simulink model "AcasXuClosedLoop_all_comp"  (Simulink 10.0)
settings: Solver=VariableStepAuto  StartTime=0.0  StopTime=20  RelTol=1e-3
libraries: neural x14040, simulink x2
(part 37 of 534)

== system AcasXuClosedLoop_all_comp/networks/Networks_m_9/AcasXu_2_9/Layer 5 ==
  "a{4} " : Inport  PortDimensions=50
      mask Display: e = 0.06;c1 = [120 230 180]/255;c2 = ([120 230 180]+0)/(256+128);c3 = ([120 230 180]+128)/(256+128);patch([0 1 1 0],[0 0 1 1],c1);patch([0 1 1 1-e 1-e 0+e],[0 0 1 1-e 0+e 0+e],c2);patch([0 0 1 1-e 0+e 0+e],[0 1 1 1-e 1-e 0+e],c3);plot([0 1 1 0 0],[0 0 1 1 0]);
  Delays 1 : Subsystem
      mask Display: e = 0.06;c1 = [120 150 230]/255;c2 = ([120 150 230]+0)/383;c3 = ([120 150 230]+128)/383;patch([0 1 1 0],[0 0 1 1],c1);patch([0 1 1 1-e 1-e 0+e],[0 0 1 1-e 0+e 0+e],c2);patch([0 0 1 1-e 0+e 0+e],[0 1 1 1-e 1-e 0+e],c3);plot([0 1 1 0 0],[0 0 1 1 0]);disp('0');
  "LW{5,4}" : Subsystem
      mask Display: e = 0.06;c1 = [120 150 230]/255;c2 = ([120 150 230]+0)/383;c3 = ([120 150 230]+128)/383;patch([0 1 1 0],[0 0 1 1],c1);patch([0 1 1 1-e 1-e 0+e],[0 0 1 1-e 0+e 0+e],c2);patch([0 0 1 1-e 0+e 0+e],[0 1 1 1-e 1-e 0+e],c3);plot([0 1 1 0 0],[0 0 1 1 0]);disp('W');
  "b{5}" : Constant  Value=[-1.912080000000000001847411112976260483264923095703125;1.2460500000000001019628825815743766725063323974609375;2.856149999999999966604491419275291264057159423828125;-1.15819999999999989626076057902537286281585693359375;-3.26635000000000008668621376273222267627716064453125;-2.868939999999999823643292984343133866786956787109375;-0.322438999999999975631936877107364125549793243408203125;-4.8585200000000003939248927053995430469512939453125;0.00826776000000000073353323415403792751021683216094970703125;1.1786600000000000409983158533577807247638702392578125;1.7016999999999999904076730672386474907398223876953125;0.33088200000000000944311295825173147022724151611328125;3.350420000000000175788272827048785984516143798828125;-3.92804999999999981952214511693455278873443603515625;0.65110299999999998732391759403981268405914306640625;2.038600000000000189714910447946749627590179443359375;0.12242699999999999416200324731107684783637523651123046875;-0.7051979999999999915161197350244037806987762451171875;1.2552600000000000424194013248779810965061187744140625;-0.79386999999999996457944462235900573432445526123046875;-0.045150900000000000755751017322836560197174549102783203125;-0.47827399999999997692867736986954696476459503173828125;-1.86057000000000005712763595511205494403839111328125;2.501650000000000151345602716901339590549468994140625;-2.96544000000000007588596417917869985103607177734375;-0.56893000000000004678923914980259723961353302001953125;-1.4840899999999999092636926434352062642574310302734375;-0.94826799999999999979394260662957094609737396240234375;-0.71766300000000005088196530778077431023120880126953125;0.272494999999999987227994324712199158966541290283203125;0.89623799999999997911714899601065553724765777587890625;3.005290000000000016910917111090384423732757568359375;0.328612999999999988443022402861970476806163787841796875;-0.63532500000000002859934511434403248131275177001953125;-4.05874000000000023646862246096134185791015625;0.52727900000000005320544005371630191802978515625;-1.0708699999999999885602619542623870074748992919921875;0.07364610000000000600284266738526639528572559356689453125;0.260485000000000022080115513745113275945186614990234375;0.558702999999999949665152598754502832889556884765625;0.1783879999999999910187398199923336505889892578125;-2.481310000000000126618715512449853122234344482421875;-3.99228000000000005087485988042317330837249755859375;0.448550999999999977507769699514028616249561309814453125;-1.1849700000000000787991893957951106131076812744140625;3.7723399999999998044586391188204288482666015625;2.011979999999999879634060562239028513431549072265625;1.1504399999999999071320644361549057066440582275390625;-4.28300000000000036237679523765109479427337646484375;-1.9330400000000000915179043659009039402008056640625]
      mask Display: e = 0.06;c1 = [120 150 230]/255;c2 = ([120 150 230]+0)/383;c3 = ([120 150 230]+128)/383;patch([0 1 1 0],[0 0 1 1],c1);patch([0 1 1 1-e 1-e 0+e],[0 0 1 1-e 0+e 0+e],c2);patch([0 0 1 1-e 0+e 0+e],[0 1 1 1-e 1-e 0+e],c3);plot([0 1 1 0 0],[0 0 1 1 0]);disp('b');
  netsum : Sum  Inputs=2  SaturateOnIntegerOverflow=on  InputSameDT=on  OutDataTypeStr=Inherit: Same as first input
      mask Display: e = 0.06;c1 = [120 150 230]/255;c2 = ([120 150 230]+0)/383;c3 = ([120 150 230]+128)/383;patch([0 1 1 0],[0 0 1 1],c1);patch([0 1 1 1-e 1-e 0+e],[0 0 1 1-e 0+e 0+e],c2);patch([0 0 1 1-e 0+e 0+e],[0 1 1 1-e 1-e 0+e],c3);plot([0 1 1 0 0],[0 0 1 1 0]);disp('+');
  poslin : neural/Transfer Functions/poslin
      settings: SourceProductName=Deep Learning Toolbox  SourceProductBaseCode=NN
  "a{5}" : Outport  InitialOutput=[0;0;0;0;0;0;0;0;0;0;0;0;0;0;0;0;0;0;0;0;0;0;0;0;0;0;0;0;0;0;0;0;0;0;0;0;0;0;0;0;0;0;0;0;0;0;0;0;0;0]
      mask Display: e = 0.06;c1 = [120 230 180]/255;c2 = ([120 230 180]+0)/(256+128);c3 = ([120 230 180]+128)/(256+128);patch([0 1 1 0],[0 0 1 1],c1);patch([0 1 1 1-e 1-e 0+e],[0 0 1 1-e 0+e 0+e],c2);patch([0 0 1 1-e 0+e 0+e],[0 1 1 1-e 1-e 0+e],c3);plot([0 1 1 0 0],[0 0 1 1 0]);
  wires:
  poslin/1 -> "a{5}"/1
  netsum/1 -> poslin/1
  "b{5}"/1 -> netsum/2
  "LW{5,4}"/1("lz{5,4}") -> netsum/1
  Delays 1/1("pd{5,4}") -> "LW{5,4}"/1("ad{5,4}")
  "a{4} "/1 -> Delays 1/1("p{5}")

== system AcasXuClosedLoop_all_comp/networks/Networks_m_9/AcasXu_2_9/Layer 6 ==
  "a{5} " : Inport  PortDimensions=50
      mask Display: e = 0.06;c1 = [120 230 180]/255;c2 = ([120 230 180]+0)/(256+128);c3 = ([120 230 180]+128)/(256+128);patch([0 1 1 0],[0 0 1 1],c1);patch([0 1 1 1-e 1-e 0+e],[0 0 1 1-e 0+e 0+e],c2);patch([0 0 1 1-e 0+e 0+e],[0 1 1 1-e 1-e 0+e],c3);plot([0 1 1 0 0],[0 0 1 1 0]);
  Delays 1 : Subsystem
      mask Display: e = 0.06;c1 = [120 150 230]/255;c2 = ([120 150 230]+0)/383;c3 = ([120 150 230]+128)/383;patch([0 1 1 0],[0 0 1 1],c1);patch([0 1 1 1-e 1-e 0+e],[0 0 1 1-e 0+e 0+e],c2);patch([0 0 1 1-e 0+e 0+e],[0 1 1 1-e 1-e 0+e],c3);plot([0 1 1 0 0],[0 0 1 1 0]);disp('0');
  "LW{6,5}" : Subsystem
      mask Display: e = 0.06;c1 = [120 150 230]/255;c2 = ([120 150 230]+0)/383;c3 = ([120 150 230]+128)/383;patch([0 1 1 0],[0 0 1 1],c1);patch([0 1 1 1-e 1-e 0+e],[0 0 1 1-e 0+e 0+e],c2);patch([0 0 1 1-e 0+e 0+e],[0 1 1 1-e 1-e 0+e],c3);plot([0 1 1 0 0],[0 0 1 1 0]);disp('W');
  "b{6}" : Constant  Value=[-0.44546200000000002461320036672987043857574462890625;0.005623999999999999659106020288845684262923896312713623046875;5.890679999999999694182406528852880001068115234375;0.277841000000000004632738637155853211879730224609375;1.4084000000000000962785406954935751855373382568359375;0.0146207999999999997686739305891023832373321056365966796875;3.39046000000000002927436071331612765789031982421875;-5.8207199999999996720134731731377542018890380859375;-7.1486400000000003274180926382541656494140625;4.2595200000000001949729266925714910030364990234375;-5.89017000000000034987124308827333152294158935546875;-6.1283200000000004337152859079651534557342529296875;0.1922750000000000014654943925052066333591938018798828125;0.1213889999999999969038100289253634400665760040283203125;-5.73660000000000014352963262354023754596710205078125;3.083650000000000002131628207280300557613372802734375;0.78354299999999998949107293810811825096607208251953125;-5.58091999999999988091303748660720884799957275390625;-1.2775000000000000799360577730112709105014801025390625;-9.1302000000000003154809746774844825267791748046875;-1.608000000000000095923269327613525092601776123046875;3.3912800000000000721911419532261788845062255859375;-3.998089999999999921698190519236959517002105712890625;1.560869999999999979678477757261134684085845947265625;-10.4304000000000005599076757789589464664459228515625;-8.982169999999999987494447850622236728668212890625;-9.91011999999999915189619059674441814422607421875;-4.880630000000000023874235921539366245269775390625;-1.470450000000000034816594052244909107685089111328125;-3.563989999999999991331378623726777732372283935546875;-4.8996399999999997731947587453760206699371337890625;-6.07057999999999964302332955412566661834716796875;1.1889600000000000168398628375143744051456451416015625;0.056920900000000003438049844817214761860668659210205078125;-3.316940000000000221547225009999237954616546630859375;-5.3012899999999998357225194922648370265960693359375;-0.0309107999999999986939780427519508521072566509246826171875;-7.2179000000000002046363078989088535308837890625;3.530860000000000109565689854207448661327362060546875;-12.779199999999999448618837050162255764007568359375;-3.3554300000000001347189027001149952411651611328125;0.11294099999999999972555286831266130320727825164794921875;3.32683000000000017593038137420080602169036865234375;-6.00711999999999957111640469520352780818939208984375;1.030790000000000095070618044701404869556427001953125;4.65157000000000042660985855036415159702301025390625;-7.62272999999999978371079123462550342082977294921875;1.97126000000000001222133505507372319698333740234375;-0.984769000000000005456968210637569427490234375;-9.0827399999999993696064848336391150951385498046875]
      mask Display: e = 0.06;c1 = [120 150 230]/255;c2 = ([120 150 230]+0)/383;c3 = ([120 150 230]+128)/383;patch([0 1 1 0],[0 0 1 1],c1);patch([0 1 1 1-e 1-e 0+e],[0 0 1 1-e 0+e 0+e],c2);patch([0 0 1 1-e 0+e 0+e],[0 1 1 1-e 1-e 0+e],c3);plot([0 1 1 0 0],[0 0 1 1 0]);disp('b');
  netsum : Sum  Inputs=2  SaturateOnIntegerOverflow=on  InputSameDT=on  OutDataTypeStr=Inherit: Same as first input
      mask Display: e = 0.06;c1 = [120 150 230]/255;c2 = ([120 150 230]+0)/383;c3 = ([120 150 230]+128)/383;patch([0 1 1 0],[0 0 1 1],c1);patch([0 1 1 1-e 1-e 0+e],[0 0 1 1-e 0+e 0+e],c2);patch([0 0 1 1-e 0+e 0+e],[0 1 1 1-e 1-e 0+e],c3);plot([0 1 1 0 0],[0 0 1 1 0]);disp('+');
  poslin : neural/Transfer Functions/poslin
      settings: SourceProductName=Deep Learning Toolbox  SourceProductBaseCode=NN
  "a{6}" : Outport  InitialOutput=[0;0;0;0;0;0;0;0;0;0;0;0;0;0;0;0;0;0;0;0;0;0;0;0;0;0;0;0;0;0;0;0;0;0;0;0;0;0;0;0;0;0;0;0;0;0;0;0;0;0]
      mask Display: e = 0.06;c1 = [120 230 180]/255;c2 = ([120 230 180]+0)/(256+128);c3 = ([120 230 180]+128)/(256+128);patch([0 1 1 0],[0 0 1 1],c1);patch([0 1 1 1-e 1-e 0+e],[0 0 1 1-e 0+e 0+e],c2);patch([0 0 1 1-e 0+e 0+e],[0 1 1 1-e 1-e 0+e],c3);plot([0 1 1 0 0],[0 0 1 1 0]);
  wires:
  poslin/1 -> "a{6}"/1
  netsum/1 -> poslin/1
  "b{6}"/1 -> netsum/2
  "LW{6,5}"/1("lz{6,5}") -> netsum/1
  Delays 1/1("pd{6,5}") -> "LW{6,5}"/1("ad{6,5}")
  "a{5} "/1 -> Delays 1/1("p{6}")

== system AcasXuClosedLoop_all_comp/networks/Networks_m_9/AcasXu_2_9/Layer 7 ==
  "a{6} " : Inport  PortDimensions=50
      mask Display: e = 0.06;c1 = [120 230 180]/255;c2 = ([120 230 180]+0)/(256+128);c3 = ([120 230 180]+128)/(256+128);patch([0 1 1 0],[0 0 1 1],c1);patch([0 1 1 1-e 1-e 0+e],[0 0 1 1-e 0+e 0+e],c2);patch([0 0 1 1-e 0+e 0+e],[0 1 1 1-e 1-e 0+e],c3);plot([0 1 1 0 0],[0 0 1 1 0]);
  Delays 1 : Subsystem
      mask Display: e = 0.06;c1 = [120 150 230]/255;c2 = ([120 150 230]+0)/383;c3 = ([120 150 230]+128)/383;patch([0 1 1 0],[0 0 1 1],c1);patch([0 1 1 1-e 1-e 0+e],[0 0 1 1-e 0+e 0+e],c2);patch([0 0 1 1-e 0+e 0+e],[0 1 1 1-e 1-e 0+e],c3);plot([0 1 1 0 0],[0 0 1 1 0]);disp('0');
  "LW{7,6}" : Subsystem
      mask Display: e = 0.06;c1 = [120 150 230]/255;c2 = ([120 150 230]+0)/383;c3 = ([120 150 230]+128)/383;patch([0 1 1 0],[0 0 1 1],c1);patch([0 1 1 1-e 1-e 0+e],[0 0 1 1-e 0+e 0+e],c2);patch([0 0 1 1-e 0+e 0+e],[0 1 1 1-e 1-e 0+e],c3);plot([0 1 1 0 0],[0 0 1 1 0]);disp('W');
  "b{7}" : Constant  Value=[-0.021078199999999998326405403759054024703800678253173828125;-0.0197138000000000000733191285462453379295766353607177734375;0.0182673999999999996102229005146000417880713939666748046875;-0.0185578999999999987025045555810720543377101421356201171875;0.0182602000000000007362555010104188113473355770111083984375]
      mask Display: e = 0.06;c1 = [120 150 230]/255;c2 = ([120 150 230]+0)/383;c3 = ([120 150 230]+128)/383;patch([0 1 1 0],[0 0 1 1],c1);patch([0 1 1 1-e 1-e 0+e],[0 0 1 1-e 0+e 0+e],c2);patch([0 0 1 1-e 0+e 0+e],[0 1 1 1-e 1-e 0+e],c3);plot([0 1 1 0 0],[0 0 1 1 0]);disp('b');
  netsum : Sum  Inputs=2  SaturateOnIntegerOverflow=on  InputSameDT=on  OutDataTypeStr=Inherit: Same as first input
      mask Display: e = 0.06;c1 = [120 150 230]/255;c2 = ([120 150 230]+0)/383;c3 = ([120 150 230]+128)/383;patch([0 1 1 0],[0 0 1 1],c1);patch([0 1 1 1-e 1-e 0+e],[0 0 1 1-e 0+e 0+e],c2);patch([0 0 1 1-e 0+e 0+e],[0 1 1 1-e 1-e 0+e],c3);plot([0 1 1 0 0],[0 0 1 1 0]);disp('+');
  purelin : neural/Transfer Functions/purelin
      settings: SourceProductName=Deep Learning Toolbox
  "a{7}" : Outport  InitialOutput=[0;0;0;0;0]
      mask Display: e = 0.06;c1 = [120 230 180]/255;c2 = ([120 230 180]+0)/(256+128);c3 = ([120 230 180]+128)/(256+128);patch([0 1 1 0],[0 0 1 1],c1);patch([0 1 1 1-e 1-e 0+e],[0 0 1 1-e 0+e 0+e],c2);patch([0 0 1 1-e 0+e 0+e],[0 1 1 1-e 1-e 0+e],c3);plot([0 1 1 0 0],[0 0 1 1 0]);
  wires:
  purelin/1 -> "a{7}"/1
  netsum/1 -> purelin/1
  "b{7}"/1 -> netsum/2
  "LW{7,6}"/1("lz{7,6}") -> netsum/1
  Delays 1/1("pd{7,6}") -> "LW{7,6}"/1("ad{7,6}")
  "a{6} "/1 -> Delays 1/1("p{7}")

== system AcasXuClosedLoop_all_comp/networks/Networks_m_9/AcasXu_2_9/Process Input 1 ==
  x : Inport  PortDimensions=5
      mask Display: e = 0.06;c1 = [120 230 180]/255;c2 = ([120 230 180]+0)/(256+128);c3 = ([120 230 180]+128)/(256+128);patch([0 1 1 0],[0 0 1 1],c1);patch([0 1 1 1-e 1-e 0+e],[0 0 1 1-e 0+e 0+e],c2);patch([0 0 1 1-e 0+e 0+e],[0 1 1 1-e 1-e 0+e],c3);plot([0 1 1 0 0],[0 0 1 1 0]);
  p : Outport  PortDimensions=5
      mask Display: e = 0.06;c1 = [120 230 180]/255;c2 = ([120 230 180]+0)/(256+128);c3 = ([120 230 180]+128)/(256+128);patch([0 1 1 0],[0 0 1 1],c1);patch([0 1 1 1-e 1-e 0+e],[0 0 1 1-e 0+e 0+e],c2);patch([0 0 1 1-e 0+e 0+e],[0 1 1 1-e 1-e 0+e],c3);plot([0 1 1 0 0],[0 0 1 1 0]);
  wires:
  x/1 -> p/1

== system AcasXuClosedLoop_all_comp/networks/Networks_m_9/AcasXu_2_9/Process Output 1 ==
  a : Inport  PortDimensions=5
      mask Display: e = 0.06;c1 = [120 230 180]/255;c2 = ([120 230 180]+0)/(256+128);c3 = ([120 230 180]+128)/(256+128);patch([0 1 1 0],[0 0 1 1],c1);patch([0 1 1 1-e 1-e 0+e],[0 0 1 1-e 0+e 0+e],c2);patch([0 0 1 1-e 0+e 0+e],[0 1 1 1-e 1-e 0+e],c3);plot([0 1 1 0 0],[0 0 1 1 0]);
  y : Outport  PortDimensions=5
      mask Display: e = 0.06;c1 = [120 230 180]/255;c2 = ([120 230 180]+0)/(256+128);c3 = ([120 230 180]+128)/(256+128);patch([0 1 1 0],[0 0 1 1],c1);patch([0 1 1 1-e 1-e 0+e],[0 0 1 1-e 0+e 0+e],c2);patch([0 0 1 1-e 0+e 0+e],[0 1 1 1-e 1-e 0+e],c3);plot([0 1 1 0 0],[0 0 1 1 0]);
  wires:
  a/1 -> y/1

== system AcasXuClosedLoop_all_comp/networks/Networks_m_9/AcasXu_3_9/Layer 1 ==
  "p{1}" : Inport  PortDimensions=5
      mask Display: e = 0.06;c1 = [120 230 180]/255;c2 = ([120 230 180]+0)/(256+128);c3 = ([120 230 180]+128)/(256+128);patch([0 1 1 0],[0 0 1 1],c1);patch([0 1 1 1-e 1-e 0+e],[0 0 1 1-e 0+e 0+e],c2);patch([0 0 1 1-e 0+e 0+e],[0 1 1 1-e 1-e 0+e],c3);plot([0 1 1 0 0],[0 0 1 1 0]);
  Delays 1 : Subsystem
      mask Display: e = 0.06;c1 = [120 150 230]/255;c2 = ([120 150 230]+0)/383;c3 = ([120 150 230]+128)/383;patch([0 1 1 0],[0 0 1 1],c1);patch([0 1 1 1-e 1-e 0+e],[0 0 1 1-e 0+e 0+e],c2);patch([0 0 1 1-e 0+e 0+e],[0 1 1 1-e 1-e 0+e],c3);plot([0 1 1 0 0],[0 0 1 1 0]);disp('0');
  "IW{1,1}" : Subsystem
      mask Display: e = 0.06;c1 = [120 150 230]/255;c2 = ([120 150 230]+0)/383;c3 = ([120 150 230]+128)/383;patch([0 1 1 0],[0 0 1 1],c1);patch([0 1 1 1-e 1-e 0+e],[0 0 1 1-e 0+e 0+e],c2);patch([0 0 1 1-e 0+e 0+e],[0 1 1 1-e 1-e 0+e],c3);plot([0 1 1 0 0],[0 0 1 1 0]);disp('W');
  "b{1}" : Constant  Value=[0.030622900000000001508038138808842631988227367401123046875;0.2441520000000000079065642921705148182809352874755859375;0.0133717999999999996585398065462868544273078441619873046875;0.028875299999999999578381704168350552208721637725830078125;-0.53588299999999999823074858795735053718090057373046875;-0.1026019999999999987583265692592249251902103424072265625;-0.6446739999999999692903429604484699666500091552734375;-0.44969999999999998863131622783839702606201171875;-0.0275753999999999999115374293978675268590450286865234375;-0.021906899999999999983923970603427733294665813446044921875;-0.01108540000000000046165293809963259263895452022552490234375;-0.7542370000000000462847538074129261076450347900390625;0.2315709999999999990638599456360680051147937774658203125;0.6522229999999999972004616211052052676677703857421875;-0.00140032999999999997753352687368533224798738956451416015625;-0.54398000000000001907807245515868999063968658447265625;-0.041275499999999999689581642314806231297552585601806640625;-0.49519000000000001904254531837068498134613037109375;-0.2935820000000000096207486421917565166950225830078125;-0.52670300000000003226574563086614944040775299072265625;-0.022206599999999999950350826338762999512255191802978515625;-0.377709999999999990194510246510617434978485107421875;0.06179679999999999895354818590931245125830173492431640625;0.2306709999999999871622691216543898917734622955322265625;-0.395853999999999983661069791196496225893497467041015625;0.0292625999999999997225330616856808774173259735107421875;0.1778720000000000023288038164537283591926097869873046875;-0.09989320000000000143725031875874265097081661224365234375;-0.5966599999999999681676854379475116729736328125;0.2298300000000000065103478164019179530441761016845703125;-0.2032980000000000064819261069715139456093311309814453125;0.110547000000000006370015626089298166334629058837890625;0.10639600000000000445954384531432879157364368438720703125;-0.1522880000000000066950889276995440013706684112548828125;-0.027030800000000000660183019363103085197508335113525390625;0.120275999999999994027888305936357937753200531005859375;0.039729300000000002113242913992507965303957462310791015625;0.10090399999999999369748593380791135132312774658203125;0.0299061000000000014320544750034969183616340160369873046875;0.1286940000000000028368418725221999920904636383056640625;-0.09250629999999999963922192591780913062393665313720703125;0.00146498000000000005819955628538764358381740748882293701171875;-0.565617000000000036408209780347533524036407470703125;-0.02543890000000000040092373865263652987778186798095703125;-0.019948899999999998577937532218129490502178668975830078125;-0.10592500000000000526245713672324200160801410675048828125;-0.390361000000000013532286402551108039915561676025390625;-0.2574980000000000046611603465862572193145751953125;-0.1269470000000000042827963397940038703382015228271484375;-0.23213200000000000500222085975110530853271484375]
      mask Display: e = 0.06;c1 = [120 150 230]/255;c2 = ([120 150 230]+0)/383;c3 = ([120 150 230]+128)/383;patch([0 1 1 0],[0 0 1 1],c1);patch([0 1 1 1-e 1-e 0+e],[0 0 1 1-e 0+e 0+e],c2);patch([0 0 1 1-e 0+e 0+e],[0 1 1 1-e 1-e 0+e],c3);plot([0 1 1 0 0],[0 0 1 1 0]);disp('b');
  netsum : Sum  Inputs=2  SaturateOnIntegerOverflow=on  InputSameDT=on  OutDataTypeStr=Inherit: Same as first input
      mask Display: e = 0.06;c1 = [120 150 230]/255;c2 = ([120 150 230]+0)/383;c3 = ([120 150 230]+128)/383;patch([0 1 1 0],[0 0 1 1],c1);patch([0 1 1 1-e 1-e 0+e],[0 0 1 1-e 0+e 0+e],c2);patch([0 0 1 1-e 0+e 0+e],[0 1 1 1-e 1-e 0+e],c3);plot([0 1 1 0 0],[0 0 1 1 0]);disp('+');
  poslin : neural/Transfer Functions/poslin
      settings: SourceProductName=Deep Learning Toolbox  SourceProductBaseCode=NN
  "a{1}" : Outport  InitialOutput=[0;0;0;0;0;0;0;0;0;0;0;0;0;0;0;0;0;0;0;0;0;0;0;0;0;0;0;0;0;0;0;0;0;0;0;0;0;0;0;0;0;0;0;0;0;0;0;0;0;0]
      mask Display: e = 0.06;c1 = [120 230 180]/255;c2 = ([120 230 180]+0)/(256+128);c3 = ([120 230 180]+128)/(256+128);patch([0 1 1 0],[0 0 1 1],c1);patch([0 1 1 1-e 1-e 0+e],[0 0 1 1-e 0+e 0+e],c2);patch([0 0 1 1-e 0+e 0+e],[0 1 1 1-e 1-e 0+e],c3);plot([0 1 1 0 0],[0 0 1 1 0]);
  wires:
  poslin/1 -> "a{1}"/1
  netsum/1 -> poslin/1
  "b{1}"/1 -> netsum/2
  "IW{1,1}"/1("iz{1,1}") -> netsum/1
  Delays 1/1("pd{1,1}") -> "IW{1,1}"/1("pd{1,1}")
  "p{1}"/1 -> Delays 1/1("p{1}")

== system AcasXuClosedLoop_all_comp/networks/Networks_m_9/AcasXu_3_9/Layer 2 ==
  "a{1} " : Inport  PortDimensions=50
      mask Display: e = 0.06;c1 = [120 230 180]/255;c2 = ([120 230 180]+0)/(256+128);c3 = ([120 230 180]+128)/(256+128);patch([0 1 1 0],[0 0 1 1],c1);patch([0 1 1 1-e 1-e 0+e],[0 0 1 1-e 0+e 0+e],c2);patch([0 0 1 1-e 0+e 0+e],[0 1 1 1-e 1-e 0+e],c3);plot([0 1 1 0 0],[0 0 1 1 0]);
  Delays 1 : Subsystem
      mask Display: e = 0.06;c1 = [120 150 230]/255;c2 = ([120 150 230]+0)/383;c3 = ([120 150 230]+128)/383;patch([0 1 1 0],[0 0 1 1],c1);patch([0 1 1 1-e 1-e 0+e],[0 0 1 1-e 0+e 0+e],c2);patch([0 0 1 1-e 0+e 0+e],[0 1 1 1-e 1-e 0+e],c3);plot([0 1 1 0 0],[0 0 1 1 0]);disp('0');
  "LW{2,1}" : Subsystem
      mask Display: e = 0.06;c1 = [120 150 230]/255;c2 = ([120 150 230]+0)/383;c3 = ([120 150 230]+128)/383;patch([0 1 1 0],[0 0 1 1],c1);patch([0 1 1 1-e 1-e 0+e],[0 0 1 1-e 0+e 0+e],c2);patch([0 0 1 1-e 0+e 0+e],[0 1 1 1-e 1-e 0+e],c3);plot([0 1 1 0 0],[0 0 1 1 0]);disp('W');
  "b{2}" : Constant  Value=[1.1304700000000000859046167533961124718189239501953125;-0.020277599999999999791366889212440582923591136932373046875;1.5884499999999999175770426518283784389495849609375;-1.934600000000000097344354799133725464344024658203125;-0.0215263000000000016831425142527223215438425540924072265625;-1.02658000000000004803268893738277256488800048828125;0.37367099999999997539390506062773056328296661376953125;-0.0209797000000000004316103030532758566550910472869873046875;0.13788400000000000655830945106572471559047698974609375;0.9806209999999999649133997081662528216838836669921875;1.7327600000000000779465381128829903900623321533203125;-0.046625199999999998590194394409991218708455562591552734375;0.290381999999999973471886960396659560501575469970703125;-0.403724000000000027288393766866647638380527496337890625;-0.0184929999999999990223376045150871505029499530792236328125;-0.0272172999999999998266275724745355546474456787109375;-0.036036800000000000776534392343819490633904933929443359375;-0.021002500000000000335287353436797275207936763763427734375;-0.032667099999999997528288275816521490924060344696044921875;-0.0185414999999999989543919554080275702290236949920654296875;-1.3001899999999999568700559393619187176227569580078125;1.0247599999999998932054268152569420635700225830078125;-0.0304184000000000016872281349833428976126015186309814453125;-0.52067600000000002768985041257110424339771270751953125;0.306744999999999989892529583812574855983257293701171875;0.2081530000000000046878056991772609762847423553466796875;-0.01226089999999999992363886036628173314966261386871337890625;-0.013461199999999999554756158204327221028506755828857421875;-0.0311606000000000001037836483419596333988010883331298828125;-0.020854799999999999726707500258271466009318828582763671875;-0.0218689000000000001444622199642253690399229526519775390625;0.89031099999999996352784137343405745923519134521484375;-0.0130860999999999998155697511492689955048263072967529296875;-0.0115549999999999992994492714615262229926884174346923828125;1.01635000000000008668621376273222267627716064453125;-0.29596699999999998009769797135959379374980926513671875;1.4970399999999999263167183016776107251644134521484375;-0.4670900000000000051869619710487313568592071533203125;-0.0189642999999999999072297640623219194822013378143310546875;-0.0109682000000000008654854610767870326526463031768798828125;-0.21932099999999998818367430430953390896320343017578125;-1.5291900000000000492406115881749428808689117431640625;-0.01226900000000000039157566078529271180741488933563232421875;-0.20906700000000000283506551568279974162578582763671875;0.74215299999999995161914512209477834403514862060546875;-0.01379310000000000084596774030387678067199885845184326171875;-0.01690679999999999960635932438890449702739715576171875;-0.37743300000000001848121655712020583450794219970703125;-0.0114754999999999995896615700985421426594257354736328125;0.738626000000000004774847184307873249053955078125]
      mask Display: e = 0.06;c1 = [120 150 230]/255;c2 = ([120 150 230]+0)/383;c3 = ([120 150 230]+128)/383;patch([0 1 1 0],[0 0 1 1],c1);patch([0 1 1 1-e 1-e 0+e],[0 0 1 1-e 0+e 0+e],c2);patch([0 0 1 1-e 0+e 0+e],[0 1 1 1-e 1-e 0+e],c3);plot([0 1 1 0 0],[0 0 1 1 0]);disp('b');
  netsum : Sum  Inputs=2  SaturateOnIntegerOverflow=on  InputSameDT=on  OutDataTypeStr=Inherit: Same as first input
      mask Display: e = 0.06;c1 = [120 150 230]/255;c2 = ([120 150 230]+0)/383;c3 = ([120 150 230]+128)/383;patch([0 1 1 0],[0 0 1 1],c1);patch([0 1 1 1-e 1-e 0+e],[0 0 1 1-e 0+e 0+e],c2);patch([0 0 1 1-e 0+e 0+e],[0 1 1 1-e 1-e 0+e],c3);plot([0 1 1 0 0],[0 0 1 1 0]);disp('+');
  poslin : neural/Transfer Functions/poslin
      settings: SourceProductName=Deep Learning Toolbox  SourceProductBaseCode=NN
  "a{2}" : Outport  InitialOutput=[0;0;0;0;0;0;0;0;0;0;0;0;0;0;0;0;0;0;0;0;0;0;0;0;0;0;0;0;0;0;0;0;0;0;0;0;0;0;0;0;0;0;0;0;0;0;0;0;0;0]
      mask Display: e = 0.06;c1 = [120 230 180]/255;c2 = ([120 230 180]+0)/(256+128);c3 = ([120 230 180]+128)/(256+128);patch([0 1 1 0],[0 0 1 1],c1);patch([0 1 1 1-e 1-e 0+e],[0 0 1 1-e 0+e 0+e],c2);patch([0 0 1 1-e 0+e 0+e],[0 1 1 1-e 1-e 0+e],c3);plot([0 1 1 0 0],[0 0 1 1 0]);
  wires:
  poslin/1 -> "a{2}"/1
  netsum/1 -> poslin/1
  "b{2}"/1 -> netsum/2
  "LW{2,1}"/1("lz{2,1}") -> netsum/1
  Delays 1/1("pd{2,1}") -> "LW{2,1}"/1("ad{2,1}")
  "a{1} "/1 -> Delays 1/1("p{2}")

== system AcasXuClosedLoop_all_comp/networks/Networks_m_9/AcasXu_3_9/Layer 3 ==
  "a{2} " : Inport  PortDimensions=50
      mask Display: e = 0.06;c1 = [120 230 180]/255;c2 = ([120 230 180]+0)/(256+128);c3 = ([120 230 180]+128)/(256+128);patch([0 1 1 0],[0 0 1 1],c1);patch([0 1 1 1-e 1-e 0+e],[0 0 1 1-e 0+e 0+e],c2);patch([0 0 1 1-e 0+e 0+e],[0 1 1 1-e 1-e 0+e],c3);plot([0 1 1 0 0],[0 0 1 1 0]);
  Delays 1 : Subsystem
      mask Display: e = 0.06;c1 = [120 150 230]/255;c2 = ([120 150 230]+0)/383;c3 = ([120 150 230]+128)/383;patch([0 1 1 0],[0 0 1 1],c1);patch([0 1 1 1-e 1-e 0+e],[0 0 1 1-e 0+e 0+e],c2);patch([0 0 1 1-e 0+e 0+e],[0 1 1 1-e 1-e 0+e],c3);plot([0 1 1 0 0],[0 0 1 1 0]);disp('0');
  "LW{3,2}" : Subsystem
      mask Display: e = 0.06;c1 = [120 150 230]/255;c2 = ([120 150 230]+0)/383;c3 = ([120 150 230]+128)/383;patch([0 1 1 0],[0 0 1 1],c1);patch([0 1 1 1-e 1-e 0+e],[0 0 1 1-e 0+e 0+e],c2);patch([0 0 1 1-e 0+e 0+e],[0 1 1 1-e 1-e 0+e],c3);plot([0 1 1 0 0],[0 0 1 1 0]);disp('W');
  "b{3}" : Constant  Value=[-0.94015199999999998770050524399266578257083892822265625;-0.57625300000000001521271997262374497950077056884765625;0.13505800000000001137578919951920397579669952392578125;-0.95997399999999999398170302811195142567157745361328125;-0.405694999999999972306596873750095255672931671142578125;0.28235799999999999787547722007730044424533843994140625;0.55059599999999997432809095698758028447628021240234375;-0.0190257999999999989626520147112387348897755146026611328125;-0.0220898999999999991195043236302808509208261966705322265625;-0.94089199999999995061017443731543608009815216064453125;0.035043100000000000637800923186659929342567920684814453125;-0.186477000000000003865352482534945011138916015625;-0.360105999999999981664444703710614703595638275146484375;-0.56810700000000002862776682377443648874759674072265625;1.3017700000000000937205868467572145164012908935546875;-0.70688099999999998157562686174060218036174774169921875;0.325824999999999975752729142186581157147884368896484375;-0.8053090000000000525659515915322117507457733154296875;-0.417132000000000002781774810500792227685451507568359375;-0.327361999999999986332710477654472924768924713134765625;-1.4274299999999999766231439934927038848400115966796875;0.052068200000000001981259600825069355778396129608154296875;-1.2055499999999998994582028899458236992359161376953125;1.8280600000000000182609483090345747768878936767578125;-0.046557700000000000473132644174256711266934871673583984375;-0.039566600000000000381117359893323737196624279022216796875;-0.7274030000000000217852402784046716988086700439453125;-1.2236100000000000864730509420041926205158233642578125;-1.490320000000000089102059064316563308238983154296875;0.09272080000000000599147398361310479231178760528564453125;0.52890000000000003677058657558518461883068084716796875;-0.9478429999999999910897940935683436691761016845703125;-0.62088799999999999545963191849295981228351593017578125;0.672177000000000024471091819577850401401519775390625;-0.6047759999999999802611228005844168365001678466796875;-1.104600000000000026290081223123706877231597900390625;-0.368530999999999997474020574372843839228153228759765625;-0.86718499999999998362198994072969071567058563232421875;-0.2483660000000000034336977705606841482222080230712890625;-0.0255894000000000017280843422895486583001911640167236328125;1.93771000000000004348521542851813137531280517578125;0.99385500000000004394706820676219649612903594970703125;-0.34159600000000001074340616469271481037139892578125;-0.006038739999999999906454828391133560216985642910003662109375;-0.2003359999999999863096178387422696687281131744384765625;0.1015790000000000026236790517941699363291263580322265625;-0.0100161000000000000031974423109204508364200592041015625;-0.5780790000000000095070618044701404869556427001953125;-0.91156800000000004491340632739593274891376495361328125;-1.043930000000000024584778657299466431140899658203125]
      mask Display: e = 0.06;c1 = [120 150 230]/255;c2 = ([120 150 230]+0)/383;c3 = ([120 150 230]+128)/383;patch([0 1 1 0],[0 0 1 1],c1);patch([0 1 1 1-e 1-e 0+e],[0 0 1 1-e 0+e 0+e],c2);patch([0 0 1 1-e 0+e 0+e],[0 1 1 1-e 1-e 0+e],c3);plot([0 1 1 0 0],[0 0 1 1 0]);disp('b');
  netsum : Sum  Inputs=2  SaturateOnIntegerOverflow=on  InputSameDT=on  OutDataTypeStr=Inherit: Same as first input
      mask Display: e = 0.06;c1 = [120 150 230]/255;c2 = ([120 150 230]+0)/383;c3 = ([120 150 230]+128)/383;patch([0 1 1 0],[0 0 1 1],c1);patch([0 1 1 1-e 1-e 0+e],[0 0 1 1-e 0+e 0+e],c2);patch([0 0 1 1-e 0+e 0+e],[0 1 1 1-e 1-e 0+e],c3);plot([0 1 1 0 0],[0 0 1 1 0]);disp('+');
  poslin : neural/Transfer Functions/poslin
      settings: SourceProductName=Deep Learning Toolbox  SourceProductBaseCode=NN
  "a{3}" : Outport  InitialOutput=[0;0;0;0;0;0;0;0;0;0;0;0;0;0;0;0;0;0;0;0;0;0;0;0;0;0;0;0;0;0;0;0;0;0;0;0;0;0;0;0;0;0;0;0;0;0;0;0;0;0]
      mask Display: e = 0.06;c1 = [120 230 180]/255;c2 = ([120 230 180]+0)/(256+128);c3 = ([120 230 180]+128)/(256+128);patch([0 1 1 0],[0 0 1 1],c1);patch([0 1 1 1-e 1-e 0+e],[0 0 1 1-e 0+e 0+e],c2);patch([0 0 1 1-e 0+e 0+e],[0 1 1 1-e 1-e 0+e],c3);plot([0 1 1 0 0],[0 0 1 1 0]);
  wires:
  poslin/1 -> "a{3}"/1
  netsum/1 -> poslin/1
  "b{3}"/1 -> netsum/2
  "LW{3,2}"/1("lz{3,2}") -> netsum/1
  Delays 1/1("pd{3,2}") -> "LW{3,2}"/1("ad{3,2}")
  "a{2} "/1 -> Delays 1/1("p{3}")

== system AcasXuClosedLoop_all_comp/networks/Networks_m_9/AcasXu_3_9/Layer 4 ==
  "a{3} " : Inport  PortDimensions=50
      mask Display: e = 0.06;c1 = [120 230 180]/255;c2 = ([120 230 180]+0)/(256+128);c3 = ([120 230 180]+128)/(256+128);patch([0 1 1 0],[0 0 1 1],c1);patch([0 1 1 1-e 1-e 0+e],[0 0 1 1-e 0+e 0+e],c2);patch([0 0 1 1-e 0+e 0+e],[0 1 1 1-e 1-e 0+e],c3);plot([0 1 1 0 0],[0 0 1 1 0]);
  Delays 1 : Subsystem
      mask Display: e = 0.06;c1 = [120 150 230]/255;c2 = ([120 150 230]+0)/383;c3 = ([120 150 230]+128)/383;patch([0 1 1 0],[0 0 1 1],c1);patch([0 1 1 1-e 1-e 0+e],[0 0 1 1-e 0+e 0+e],c2);patch([0 0 1 1-e 0+e 0+e],[0 1 1 1-e 1-e 0+e],c3);plot([0 1 1 0 0],[0 0 1 1 0]);disp('0');
  "LW{4,3}" : Subsystem
      mask Display: e = 0.06;c1 = [120 150 230]/255;c2 = ([120 150 230]+0)/383;c3 = ([120 150 230]+128)/383;patch([0 1 1 0],[0 0 1 1],c1);patch([0 1 1 1-e 1-e 0+e],[0 0 1 1-e 0+e 0+e],c2);patch([0 0 1 1-e 0+e 0+e],[0 1 1 1-e 1-e 0+e],c3);plot([0 1 1 0 0],[0 0 1 1 0]);disp('W');
  "b{4}" : Constant  Value=[-1.7806699999999999750599499748204834759235382080078125;-1.37105999999999994543031789362430572509765625;-2.34382000000000001449507180950604379177093505859375;0.50524299999999999766231439934927038848400115966796875;-3.99293999999999993377741702715866267681121826171875;3.939529999999999976267872625612653791904449462890625;-1.6768400000000001082156586562632583081722259521484375;-0.021833499999999998741895268494772608391940593719482421875;1.2009600000000000274980038739158771932125091552734375;0.41558499999999998220090446920949034392833709716796875;3.70685999999999982179588187136687338352203369140625;-1.44794000000000000483169060316868126392364501953125;1.147559999999999913455894784419797360897064208984375;-1.581539999999999945856643535080365836620330810546875;1.3639900000000000357402996087330393493175506591796875;0.786737000000000019639401216409169137477874755859375;-3.29525999999999985590193318785168230533599853515625;-0.0174671999999999986996623846380316535942256450653076171875;0.2804729999999999723314658695016987621784210205078125;-2.61798000000000019582557797548361122608184814453125;0.435118999999999978012255041903699748218059539794921875;0.922158000000000033224978324142284691333770751953125;-1.2847999999999999420907670355518348515033721923828125;1.555839999999999889723767410032451152801513671875;3.292829999999999923687710179365240037441253662109375;-0.615458999999999978314235704601742327213287353515625;1.085129999999999927950966593925841152667999267578125;-0.2290149999999999963495866950324852950870990753173828125;-1.8910899999999999376854020738392136991024017333984375;-0.7731500000000000039079850466805510222911834716796875;-0.97648900000000005139355607752804644405841827392578125;0.426566999999999973969266875428729690611362457275390625;-2.37408999999999981156406647642143070697784423828125;-1.9548200000000000020605739337042905390262603759765625;1.6051800000000000512301312483032234013080596923828125;4.26851999999999964785502015729434788227081298828125;0.470463000000000020062174144186428748071193695068359375;-0.07553169999999999328910149642979376949369907379150390625;0.5801410000000000177777792487177066504955291748046875;1.7715899999999999980815346134477294981479644775390625;-2.001310000000000144382283906452357769012451171875;-0.006227690000000000135338407147855832590721547603607177734375;-1.2850200000000000510880227011512033641338348388671875;1.6148800000000000931521526581491343677043914794921875;-1.2901499999999999079847157190670259296894073486328125;0.1793760000000000076614270483332802541553974151611328125;-0.43104500000000001147526518252561800181865692138671875;0.0135645999999999995744293102006849949248135089874267578125;-0.62482300000000001727329390632803551852703094482421875;-0.006054269999999999617157353526408769539557397365570068359375]
      mask Display: e = 0.06;c1 = [120 150 230]/255;c2 = ([120 150 230]+0)/383;c3 = ([120 150 230]+128)/383;patch([0 1 1 0],[0 0 1 1],c1);patch([0 1 1 1-e 1-e 0+e],[0 0 1 1-e 0+e 0+e],c2);patch([0 0 1 1-e 0+e 0+e],[0 1 1 1-e 1-e 0+e],c3);plot([0 1 1 0 0],[0 0 1 1 0]);disp('b');
  netsum : Sum  Inputs=2  SaturateOnIntegerOverflow=on  InputSameDT=on  OutDataTypeStr=Inherit: Same as first input
      mask Display: e = 0.06;c1 = [120 150 230]/255;c2 = ([120 150 230]+0)/383;c3 = ([120 150 230]+128)/383;patch([0 1 1 0],[0 0 1 1],c1);patch([0 1 1 1-e 1-e 0+e],[0 0 1 1-e 0+e 0+e],c2);patch([0 0 1 1-e 0+e 0+e],[0 1 1 1-e 1-e 0+e],c3);plot([0 1 1 0 0],[0 0 1 1 0]);disp('+');
  poslin : neural/Transfer Functions/poslin
      settings: SourceProductName=Deep Learning Toolbox  SourceProductBaseCode=NN
  "a{4}" : Outport  InitialOutput=[0;0;0;0;0;0;0;0;0;0;0;0;0;0;0;0;0;0;0;0;0;0;0;0;0;0;0;0;0;0;0;0;0;0;0;0;0;0;0;0;0;0;0;0;0;0;0;0;0;0]
      mask Display: e = 0.06;c1 = [120 230 180]/255;c2 = ([120 230 180]+0)/(256+128);c3 = ([120 230 180]+128)/(256+128);patch([0 1 1 0],[0 0 1 1],c1);patch([0 1 1 1-e 1-e 0+e],[0 0 1 1-e 0+e 0+e],c2);patch([0 0 1 1-e 0+e 0+e],[0 1 1 1-e 1-e 0+e],c3);plot([0 1 1 0 0],[0 0 1 1 0]);
  wires:
  poslin/1 -> "a{4}"/1
  netsum/1 -> poslin/1
  "b{4}"/1 -> netsum/2
  "LW{4,3}"/1("lz{4,3}") -> netsum/1
  Delays 1/1("pd{4,3}") -> "LW{4,3}"/1("ad{4,3}")
  "a{3} "/1 -> Delays 1/1("p{4}")

== system AcasXuClosedLoop_all_comp/networks/Networks_m_9/AcasXu_3_9/Layer 5 ==
  "a{4} " : Inport  PortDimensions=50
      mask Display: e = 0.06;c1 = [120 230 180]/255;c2 = ([120 230 180]+0)/(256+128);c3 = ([120 230 180]+128)/(256+128);patch([0 1 1 0],[0 0 1 1],c1);patch([0 1 1 1-e 1-e 0+e],[0 0 1 1-e 0+e 0+e],c2);patch([0 0 1 1-e 0+e 0+e],[0 1 1 1-e 1-e 0+e],c3);plot([0 1 1 0 0],[0 0 1 1 0]);
  Delays 1 : Subsystem
      mask Display: e = 0.06;c1 = [120 150 230]/255;c2 = ([120 150 230]+0)/383;c3 = ([120 150 230]+128)/383;patch([0 1 1 0],[0 0 1 1],c1);patch([0 1 1 1-e 1-e 0+e],[0 0 1 1-e 0+e 0+e],c2);patch([0 0 1 1-e 0+e 0+e],[0 1 1 1-e 1-e 0+e],c3);plot([0 1 1 0 0],[0 0 1 1 0]);disp('0');
  "LW{5,4}" : Subsystem
      mask Display: e = 0.06;c1 = [120 150 230]/255;c2 = ([120 150 230]+0)/383;c3 = ([120 150 230]+128)/383;patch([0 1 1 0],[0 0 1 1],c1);patch([0 1 1 1-e 1-e 0+e],[0 0 1 1-e 0+e 0+e],c2);patch([0 0 1 1-e 0+e 0+e],[0 1 1 1-e 1-e 0+e],c3);plot([0 1 1 0 0],[0 0 1 1 0]);disp('W');
  "b{5}" : Constant  Value=[-0.88253000000000003666400516522116959095001220703125;-1.040929999999999910897940935683436691761016845703125;0.324892000000000014114931445874390192329883575439453125;1.6505700000000000926547727431170642375946044921875;-1.3145000000000000017763568394002504646778106689453125;-4.08518000000000025551116777933202683925628662109375;-1.489689999999999958646412778762169182300567626953125;2.189340000000000063806737671256996691226959228515625;-3.399620000000000086259888121276162564754486083984375;0.81548699999999996190780393590102903544902801513671875;-1.3623700000000000809308176030754111707210540771484375;-1.3707300000000000039790393202565610408782958984375;-0.991616000000000052949644668842665851116180419921875;2.801790000000000002700062395888380706310272216796875;0.2169310000000000127062804722299915738403797149658203125;-0.047221800000000001273914307375889620743691921234130859375;0.60263699999999997825028685838333331048488616943359375;0.56045000000000000373034936274052597582340240478515625;0.1636249999999999926725280374739668332040309906005859375;1.39562000000000008270717444247566163539886474609375;2.188079999999999802895445100148208439350128173828125;-1.2568999999999999062083588796667754650115966796875;-2.22595000000000009521272659185342490673065185546875;-1.3807700000000000528643795405514538288116455078125;-0.00805607000000000024464430481430099462158977985382080078125;-2.13529999999999997584154698415659368038177490234375;4.42799000000000031462832339457236230373382568359375;-1.04276999999999997470467860694043338298797607421875;-1.6476900000000000989786030913819558918476104736328125;-0.7513769999999999615880597048089839518070220947265625;0.45278899999999999703703679188038222491741180419921875;-0.86635899999999999021582652858342044055461883544921875;0.932158999999999959840124574839137494564056396484375;2.6023399999999998755129126948304474353790283203125;0.0573641000000000012004619520666892640292644500732421875;-1.455179999999999918003368293284438550472259521484375;-0.029614700000000000745803419022195157594978809356689453125;1.064130000000000020321522242738865315914154052734375;0.64470799999999994778221434899023734033107757568359375;3.64191999999999982406961862579919397830963134765625;0.33077400000000001245581415787455625832080841064453125;-0.74470300000000000384403620046214200556278228759765625;0.063094700000000003559108563422341831028461456298828125;0.1389110000000000066933125708601437509059906005859375;-1.7945599999999999329958200178225524723529815673828125;-0.51627199999999995316812828605179674923419952392578125;1.1085599999999999898392388786305673420429229736328125;1.22980000000000000426325641456060111522674560546875;-1.1951300000000000256505927609396167099475860595703125;0.289219999999999977102760340130771510303020477294921875]
      mask Display: e = 0.06;c1 = [120 150 230]/255;c2 = ([120 150 230]+0)/383;c3 = ([120 150 230]+128)/383;patch([0 1 1 0],[0 0 1 1],c1);patch([0 1 1 1-e 1-e 0+e],[0 0 1 1-e 0+e 0+e],c2);patch([0 0 1 1-e 0+e 0+e],[0 1 1 1-e 1-e 0+e],c3);plot([0 1 1 0 0],[0 0 1 1 0]);disp('b');
  netsum : Sum  Inputs=2  SaturateOnIntegerOverflow=on  InputSameDT=on  OutDataTypeStr=Inherit: Same as first input
      mask Display: e = 0.06;c1 = [120 150 230]/255;c2 = ([120 150 230]+0)/383;c3 = ([120 150 230]+128)/383;patch([0 1 1 0],[0 0 1 1],c1);patch([0 1 1 1-e 1-e 0+e],[0 0 1 1-e 0+e 0+e],c2);patch([0 0 1 1-e 0+e 0+e],[0 1 1 1-e 1-e 0+e],c3);plot([0 1 1 0 0],[0 0 1 1 0]);disp('+');
  poslin : neural/Transfer Functions/poslin
      settings: SourceProductName=Deep Learning Toolbox  SourceProductBaseCode=NN
  "a{5}" : Outport  InitialOutput=[0;0;0;0;0;0;0;0;0;0;0;0;0;0;0;0;0;0;0;0;0;0;0;0;0;0;0;0;0;0;0;0;0;0;0;0;0;0;0;0;0;0;0;0;0;0;0;0;0;0]
      mask Display: e = 0.06;c1 = [120 230 180]/255;c2 = ([120 230 180]+0)/(256+128);c3 = ([120 230 180]+128)/(256+128);patch([0 1 1 0],[0 0 1 1],c1);patch([0 1 1 1-e 1-e 0+e],[0 0 1 1-e 0+e 0+e],c2);patch([0 0 1 1-e 0+e 0+e],[0 1 1 1-e 1-e 0+e],c3);plot([0 1 1 0 0],[0 0 1 1 0]);
  wires:
  poslin/1 -> "a{5}"/1
  netsum/1 -> poslin/1
  "b{5}"/1 -> netsum/2
  "LW{5,4}"/1("lz{5,4}") -> netsum/1
  Delays 1/1("pd{5,4}") -> "LW{5,4}"/1("ad{5,4}")
  "a{4} "/1 -> Delays 1/1("p{5}")

== system AcasXuClosedLoop_all_comp/networks/Networks_m_9/AcasXu_3_9/Layer 6 ==
  "a{5} " : Inport  PortDimensions=50
      mask Display: e = 0.06;c1 = [120 230 180]/255;c2 = ([120 230 180]+0)/(256+128);c3 = ([120 230 180]+128)/(256+128);patch([0 1 1 0],[0 0 1 1],c1);patch([0 1 1 1-e 1-e 0+e],[0 0 1 1-e 0+e 0+e],c2);patch([0 0 1 1-e 0+e 0+e],[0 1 1 1-e 1-e 0+e],c3);plot([0 1 1 0 0],[0 0 1 1 0]);
  Delays 1 : Subsystem
      mask Display: e = 0.06;c1 = [120 150 230]/255;c2 = ([120 150 230]+0)/383;c3 = ([120 150 230]+128)/383;patch([0 1 1 0],[0 0 1 1],c1);patch([0 1 1 1-e 1-e 0+e],[0 0 1 1-e 0+e 0+e],c2);patch([0 0 1 1-e 0+e 0+e],[0 1 1 1-e 1-e 0+e],c3);plot([0 1 1 0 0],[0 0 1 1 0]);disp('0');
  "LW{6,5}" : Subsystem
      mask Display: e = 0.06;c1 = [120 150 230]/255;c2 = ([120 150 230]+0)/383;c3 = ([120 150 230]+128)/383;patch([0 1 1 0],[0 0 1 1],c1);patch([0 1 1 1-e 1-e 0+e],[0 0 1 1-e 0+e 0+e],c2);patch([0 0 1 1-e 0+e 0+e],[0 1 1 1-e 1-e 0+e],c3);plot([0 1 1 0 0],[0 0 1 1 0]);disp('W');
  "b{6}" : Constant  Value=[-5.20418999999999964956032272311858832836151123046875;1.465079999999999937898564894567243754863739013671875;2.796170000000000044337866711430251598358154296875;-0.1965590000000000114876996804014197550714015960693359375;-5.49082000000000025607960196794010698795318603515625;1.784909999999999996589394868351519107818603515625;0.77308699999999996865795992562198080122470855712890625;-2.87788000000000021572077457676641643047332763671875;-0.376346000000000013852030633643153123557567596435546875;-4.3456700000000001438138497178442776203155517578125;-0.0310221000000000003693489958322970778681337833404541015625;-4.00438000000000027256419343757443130016326904296875;0.35609699999999999686650653529795818030834197998046875;0.468208000000000013063328196949441917240619659423828125;-6.14893000000000000682121026329696178436279296875;1.3921799999999999730704303146922029554843902587890625;-6.22140999999999966263430906110443174839019775390625;-1.868279999999999940740735837607644498348236083984375;0.479810000000000014264145420384011231362819671630859375;-1.118749999999999911182158029987476766109466552734375;-0.75030300000000005322675633578910492360591888427734375;-1.811500000000000110134124042815528810024261474609375;-0.0266857999999999991047605618632587720640003681182861328125;-6.193130000000000023874235921539366245269775390625;-8.06054999999999921556081972084939479827880859375;-5.26635000000000008668621376273222267627716064453125;1.204299999999999926103555480949580669403076171875;7.5626400000000000289901436190120875835418701171875;0.171947999999999989739762895624153316020965576171875;-6.39839000000000002188471626141108572483062744140625;-0.794113999999999986556531439418904483318328857421875;1.5262599999999999500488456760649569332599639892578125;-4.14369999999999993889332472463138401508331298828125;0.710474000000000049936943469219841063022613525390625;-6.12783999999999995367261362844146788120269775390625;-5.95999999999999996447286321199499070644378662109375;-4.25372000000000038966163629083894193172454833984375;-3.630250000000000198951966012828052043914794921875;-5.6719600000000003348077370901592075824737548828125;-0.487281999999999992922994351829402148723602294921875;-3.81524000000000018673063095775432884693145751953125;4.90758000000000027540636438061483204364776611328125;-5.47989999999999977120523908524774014949798583984375;-3.157059999999999977404741002828814089298248291015625;-4.897149999999999891997504164464771747589111328125;-5.609239999999999781721271574497222900390625;0.28050900000000000833466629046597518026828765869140625;1.0930899999999998950528379282332025468349456787109375;-7.02667000000000019355184122105129063129425048828125;0.67298100000000005138645065017044544219970703125]
      mask Display: e = 0.06;c1 = [120 150 230]/255;c2 = ([120 150 230]+0)/383;c3 = ([120 150 230]+128)/383;patch([0 1 1 0],[0 0 1 1],c1);patch([0 1 1 1-e 1-e 0+e],[0 0 1 1-e 0+e 0+e],c2);patch([0 0 1 1-e 0+e 0+e],[0 1 1 1-e 1-e 0+e],c3);plot([0 1 1 0 0],[0 0 1 1 0]);disp('b');
  netsum : Sum  Inputs=2  SaturateOnIntegerOverflow=on  InputSameDT=on  OutDataTypeStr=Inherit: Same as first input
      mask Display: e = 0.06;c1 = [120 150 230]/255;c2 = ([120 150 230]+0)/383;c3 = ([120 150 230]+128)/383;patch([0 1 1 0],[0 0 1 1],c1);patch([0 1 1 1-e 1-e 0+e],[0 0 1 1-e 0+e 0+e],c2);patch([0 0 1 1-e 0+e 0+e],[0 1 1 1-e 1-e 0+e],c3);plot([0 1 1 0 0],[0 0 1 1 0]);disp('+');
  poslin : neural/Transfer Functions/poslin
      settings: SourceProductName=Deep Learning Toolbox  SourceProductBaseCode=NN
  "a{6}" : Outport  InitialOutput=[0;0;0;0;0;0;0;0;0;0;0;0;0;0;0;0;0;0;0;0;0;0;0;0;0;0;0;0;0;0;0;0;0;0;0;0;0;0;0;0;0;0;0;0;0;0;0;0;0;0]
      mask Display: e = 0.06;c1 = [120 230 180]/255;c2 = ([120 230 180]+0)/(256+128);c3 = ([120 230 180]+128)/(256+128);patch([0 1 1 0],[0 0 1 1],c1);patch([0 1 1 1-e 1-e 0+e],[0 0 1 1-e 0+e 0+e],c2);patch([0 0 1 1-e 0+e 0+e],[0 1 1 1-e 1-e 0+e],c3);plot([0 1 1 0 0],[0 0 1 1 0]);
  wires:
  poslin/1 -> "a{6}"/1
  netsum/1 -> poslin/1
  "b{6}"/1 -> netsum/2
  "LW{6,5}"/1("lz{6,5}") -> netsum/1
  Delays 1/1("pd{6,5}") -> "LW{6,5}"/1("ad{6,5}")
  "a{5} "/1 -> Delays 1/1("p{6}")

== system AcasXuClosedLoop_all_comp/networks/Networks_m_9/AcasXu_3_9/Layer 7 ==
  "a{6} " : Inport  PortDimensions=50
      mask Display: e = 0.06;c1 = [120 230 180]/255;c2 = ([120 230 180]+0)/(256+128);c3 = ([120 230 180]+128)/(256+128);patch([0 1 1 0],[0 0 1 1],c1);patch([0 1 1 1-e 1-e 0+e],[0 0 1 1-e 0+e 0+e],c2);patch([0 0 1 1-e 0+e 0+e],[0 1 1 1-e 1-e 0+e],c3);plot([0 1 1 0 0],[0 0 1 1 0]);
  Delays 1 : Subsystem
      mask Display: e = 0.06;c1 = [120 150 230]/255;c2 = ([120 150 230]+0)/383;c3 = ([120 150 230]+128)/383;patch([0 1 1 0],[0 0 1 1],c1);patch([0 1 1 1-e 1-e 0+e],[0 0 1 1-e 0+e 0+e],c2);patch([0 0 1 1-e 0+e 0+e],[0 1 1 1-e 1-e 0+e],c3);plot([0 1 1 0 0],[0 0 1 1 0]);disp('0');
  "LW{7,6}" : Subsystem
      mask Display: e = 0.06;c1 = [120 150 230]/255;c2 = ([120 150 230]+0)/383;c3 = ([120 150 230]+128)/383;patch([0 1 1 0],[0 0 1 1],c1);patch([0 1 1 1-e 1-e 0+e],[0 0 1 1-e 0+e 0+e],c2);patch([0 0 1 1-e 0+e 0+e],[0 1 1 1-e 1-e 0+e],c3);plot([0 1 1 0 0],[0 0 1 1 0]);disp('W');
  "b{7}" : Constant  Value=[-0.002936629999999999969528818866137953591533005237579345703125;0.020852400000000000102051700423544389195740222930908203125;-0.0188574999999999992628119116488960571587085723876953125;0.0218463999999999984591436685832377406768500804901123046875;-0.01484889999999999994628741006863492657430469989776611328125]
      mask Display: e = 0.06;c1 = [120 150 230]/255;c2 = ([120 150 230]+0)/383;c3 = ([120 150 230]+128)/383;patch([0 1 1 0],[0 0 1 1],c1);patch([0 1 1 1-e 1-e 0+e],[0 0 1 1-e 0+e 0+e],c2);patch([0 0 1 1-e 0+e 0+e],[0 1 1 1-e 1-e 0+e],c3);plot([0 1 1 0 0],[0 0 1 1 0]);disp('b');
  netsum : Sum  Inputs=2  SaturateOnIntegerOverflow=on  InputSameDT=on  OutDataTypeStr=Inherit: Same as first input
      mask Display: e = 0.06;c1 = [120 150 230]/255;c2 = ([120 150 230]+0)/383;c3 = ([120 150 230]+128)/383;patch([0 1 1 0],[0 0 1 1],c1);patch([0 1 1 1-e 1-e 0+e],[0 0 1 1-e 0+e 0+e],c2);patch([0 0 1 1-e 0+e 0+e],[0 1 1 1-e 1-e 0+e],c3);plot([0 1 1 0 0],[0 0 1 1 0]);disp('+');
  purelin : neural/Transfer Functions/purelin
      settings: SourceProductName=Deep Learning Toolbox
  "a{7}" : Outport  InitialOutput=[0;0;0;0;0]
      mask Display: e = 0.06;c1 = [120 230 180]/255;c2 = ([120 230 180]+0)/(256+128);c3 = ([120 230 180]+128)/(256+128);patch([0 1 1 0],[0 0 1 1],c1);patch([0 1 1 1-e 1-e 0+e],[0 0 1 1-e 0+e 0+e],c2);patch([0 0 1 1-e 0+e 0+e],[0 1 1 1-e 1-e 0+e],c3);plot([0 1 1 0 0],[0 0 1 1 0]);
  wires:
  purelin/1 -> "a{7}"/1
  netsum/1 -> purelin/1
  "b{7}"/1 -> netsum/2
  "LW{7,6}"/1("lz{7,6}") -> netsum/1
  Delays 1/1("pd{7,6}") -> "LW{7,6}"/1("ad{7,6}")
  "a{6} "/1 -> Delays 1/1("p{7}")

== system AcasXuClosedLoop_all_comp/networks/Networks_m_9/AcasXu_3_9/Process Input 1 ==
  x : Inport  PortDimensions=5
      mask Display: e = 0.06;c1 = [120 230 180]/255;c2 = ([120 230 180]+0)/(256+128);c3 = ([120 230 180]+128)/(256+128);patch([0 1 1 0],[0 0 1 1],c1);patch([0 1 1 1-e 1-e 0+e],[0 0 1 1-e 0+e 0+e],c2);patch([0 0 1 1-e 0+e 0+e],[0 1 1 1-e 1-e 0+e],c3);plot([0 1 1 0 0],[0 0 1 1 0]);
  p : Outport  PortDimensions=5
      mask Display: e = 0.06;c1 = [120 230 180]/255;c2 = ([120 230 180]+0)/(256+128);c3 = ([120 230 180]+128)/(256+128);patch([0 1 1 0],[0 0 1 1],c1);patch([0 1 1 1-e 1-e 0+e],[0 0 1 1-e 0+e 0+e],c2);patch([0 0 1 1-e 0+e 0+e],[0 1 1 1-e 1-e 0+e],c3);plot([0 1 1 0 0],[0 0 1 1 0]);
  wires:
  x/1 -> p/1
